simulink model "AcasXuClosedLoop_all_comp2017b"  (Simulink 9.0)
settings: Solver=VariableStepAuto  StartTime=0.0  StopTime=20  RelTol=1e-3
libraries: neural x14040, simulink x2
(part 26 of 534)

== system AcasXuClosedLoop_all_comp2017b/networks/Networks_m_6/AcasXu_2_6/Process Input 1 ==
  x : Inport  PortDimensions=5
      mask Display: e = 0.06;c1 = [120 230 180]/255;c2 = ([120 230 180]+0)/(256+128);c3 = ([120 230 180]+128)/(256+128);patch([0 1 1 0],[0 0 1 1],c1);patch([0 1 1 1-e 1-e 0+e],[0 0 1 1-e 0+e 0+e],c2);patch([0 0 1 1-e 0+e 0+e],[0 1 1 1-e 1-e 0+e],c3);plot([0 1 1 0 0],[0 0 1 1 0]);
  p : Outport  PortDimensions=5
      mask Display: e = 0.06;c1 = [120 230 180]/255;c2 = ([120 230 180]+0)/(256+128);c3 = ([120 230 180]+128)/(256+128);patch([0 1 1 0],[0 0 1 1],c1);patch([0 1 1 1-e 1-e 0+e],[0 0 1 1-e 0+e 0+e],c2);patch([0 0 1 1-e 0+e 0+e],[0 1 1 1-e 1-e 0+e],c3);plot([0 1 1 0 0],[0 0 1 1 0]);
  wires:
  x/1 -> p/1

== system AcasXuClosedLoop_all_comp2017b/networks/Networks_m_6/AcasXu_2_6/Process Output 1 ==
  a : Inport  PortDimensions=5
      mask Display: e = 0.06;c1 = [120 230 180]/255;c2 = ([120 230 180]+0)/(256+128);c3 = ([120 230 180]+128)/(256+128);patch([0 1 1 0],[0 0 1 1],c1);patch([0 1 1 1-e 1-e 0+e],[0 0 1 1-e 0+e 0+e],c2);patch([0 0 1 1-e 0+e 0+e],[0 1 1 1-e 1-e 0+e],c3);plot([0 1 1 0 0],[0 0 1 1 0]);
  y : Outport  PortDimensions=5
      mask Display: e = 0.06;c1 = [120 230 180]/255;c2 = ([120 230 180]+0)/(256+128);c3 = ([120 230 180]+128)/(256+128);patch([0 1 1 0],[0 0 1 1],c1);patch([0 1 1 1-e 1-e 0+e],[0 0 1 1-e 0+e 0+e],c2);patch([0 0 1 1-e 0+e 0+e],[0 1 1 1-e 1-e 0+e],c3);plot([0 1 1 0 0],[0 0 1 1 0]);
  wires:
  a/1 -> y/1

== system AcasXuClosedLoop_all_comp2017b/networks/Networks_m_6/AcasXu_3_6/Layer 1 ==
  "p{1}" : Inport  PortDimensions=5
      mask Display: e = 0.06;c1 = [120 230 180]/255;c2 = ([120 230 180]+0)/(256+128);c3 = ([120 230 180]+128)/(256+128);patch([0 1 1 0],[0 0 1 1],c1);patch([0 1 1 1-e 1-e 0+e],[0 0 1 1-e 0+e 0+e],c2);patch([0 0 1 1-e 0+e 0+e],[0 1 1 1-e 1-e 0+e],c3);plot([0 1 1 0 0],[0 0 1 1 0]);
  Delays 1 : Subsystem
      mask Display: e = 0.06;c1 = [120 150 230]/255;c2 = ([120 150 230]+0)/383;c3 = ([120 150 230]+128)/383;patch([0 1 1 0],[0 0 1 1],c1);patch([0 1 1 1-e 1-e 0+e],[0 0 1 1-e 0+e 0+e],c2);patch([0 0 1 1-e 0+e 0+e],[0 1 1 1-e 1-e 0+e],c3);plot([0 1 1 0 0],[0 0 1 1 0]);disp('0');
  "IW{1,1}" : Subsystem
      mask Display: e = 0.06;c1 = [120 150 230]/255;c2 = ([120 150 230]+0)/383;c3 = ([120 150 230]+128)/383;patch([0 1 1 0],[0 0 1 1],c1);patch([0 1 1 1-e 1-e 0+e],[0 0 1 1-e 0+e 0+e],c2);patch([0 0 1 1-e 0+e 0+e],[0 1 1 1-e 1-e 0+e],c3);plot([0 1 1 0 0],[0 0 1 1 0]);disp('W');
  "b{1}" : Constant  Value=[-0.04478279999999999760884605848332284949719905853271484375;-0.1006650000000000044764192352886311709880828857421875;-0.1662289999999999878799172847720910795032978057861328125;-0.114005999999999996230570786792668513953685760498046875;0.2104149999999999909316983348617213778197765350341796875;-0.1818849999999999911270975871957489289343357086181640625;-0.5026509999999999589448407277814112603664398193359375;0.7021830000000000016058265828178264200687408447265625;-0.270768999999999981920240088584250770509243011474609375;-0.09438190000000000468016736476783989928662776947021484375;0.0603599999999999969890751572165754623711109161376953125;-0.3025619999999999976125764078460633754730224609375;-0.05036960000000000048370196736868820153176784515380859375;0.01067079999999999938287142953186048544012010097503662109375;0.1083550000000000068656191842819680459797382354736328125;0.037607200000000000350564022255639429204165935516357421875;0.191655999999999993033128475872217677533626556396484375;-0.0214969999999999987483345620375985163263976573944091796875;0.08847919999999999396411709540188894607126712799072265625;-0.021716800000000001380495717739904648624360561370849609375;-0.4216670000000000140261136039043776690959930419921875;-0.300678000000000000824229573481716215610504150390625;-0.028699200000000001098410251643144874833524227142333984375;0.216254000000000001779909553079050965607166290283203125;-0.2151510000000000089048768359134555794298648834228515625;0.0309653000000000011571188451853231526911258697509765625;0.1150130000000000041193715105691808275878429412841796875;-0.2091349999999999875743839083952479995787143707275390625;0.0096367299999999996018562598010248620994389057159423828125;-0.67237700000000000244426701101474463939666748046875;-0.0248868999999999999939159778250541421584784984588623046875;0.135927999999999993274713006030651740729808807373046875;-0.380010000000000014441781104324036277830600738525390625;-0.0255956999999999990080823408789001405239105224609375;0.007737909999999999753617085929136010236106812953948974609375;-0.265739000000000002987832203871221281588077545166015625;0.1178990000000000037960745657983352430164813995361328125;-0.2221829999999999916138193611914175562560558319091796875;0.1361300000000000010036416142611415125429630279541015625;-0.0134143000000000005289546578524095821194350719451904296875;-0.1725250000000000116795462190566468052566051483154296875;0.05111580000000000290061308305666898377239704132080078125;-0.0088401000000000000411670697531008045189082622528076171875;-0.569296000000000024243718144134618341922760009765625;-0.227851999999999998980371174184256233274936676025390625;-0.407177999999999984392928809029399417340755462646484375;0.1075730000000000019522161665008752606809139251708984375;-0.28299099999999999255351212923415005207061767578125;-0.3689970000000000194262383956811390817165374755859375;0.038547199999999996744559638273130985908210277557373046875]
      mask Display: e = 0.06;c1 = [120 150 230]/255;c2 = ([120 150 230]+0)/383;c3 = ([120 150 230]+128)/383;patch([0 1 1 0],[0 0 1 1],c1);patch([0 1 1 1-e 1-e 0+e],[0 0 1 1-e 0+e 0+e],c2);patch([0 0 1 1-e 0+e 0+e],[0 1 1 1-e 1-e 0+e],c3);plot([0 1 1 0 0],[0 0 1 1 0]);disp('b');
  netsum : Sum  Inputs=2  SaturateOnIntegerOverflow=on  InputSameDT=on  OutDataTypeStr=Inherit: Same as first input
      mask Display: e = 0.06;c1 = [120 150 230]/255;c2 = ([120 150 230]+0)/383;c3 = ([120 150 230]+128)/383;patch([0 1 1 0],[0 0 1 1],c1);patch([0 1 1 1-e 1-e 0+e],[0 0 1 1-e 0+e 0+e],c2);patch([0 0 1 1-e 0+e 0+e],[0 1 1 1-e 1-e 0+e],c3);plot([0 1 1 0 0],[0 0 1 1 0]);disp('+');
  poslin : neural/Transfer Functions/poslin
      settings: SourceProductName=Deep Learning Toolbox  SourceProductBaseCode=NN
  "a{1}" : Outport  InitialOutput=[0;0;0;0;0;0;0;0;0;0;0;0;0;0;0;0;0;0;0;0;0;0;0;0;0;0;0;0;0;0;0;0;0;0;0;0;0;0;0;0;0;0;0;0;0;0;0;0;0;0]
      mask Display: e = 0.06;c1 = [120 230 180]/255;c2 = ([120 230 180]+0)/(256+128);c3 = ([120 230 180]+128)/(256+128);patch([0 1 1 0],[0 0 1 1],c1);patch([0 1 1 1-e 1-e 0+e],[0 0 1 1-e 0+e 0+e],c2);patch([0 0 1 1-e 0+e 0+e],[0 1 1 1-e 1-e 0+e],c3);plot([0 1 1 0 0],[0 0 1 1 0]);
  wires:
  poslin/1 -> "a{1}"/1
  netsum/1 -> poslin/1
  "b{1}"/1 -> netsum/2
  "IW{1,1}"/1("iz{1,1}") -> netsum/1
  Delays 1/1("pd{1,1}") -> "IW{1,1}"/1("pd{1,1}")
  "p{1}"/1 -> Delays 1/1("p{1}")

== system AcasXuClosedLoop_all_comp2017b/networks/Networks_m_6/AcasXu_3_6/Layer 2 ==
  "a{1} " : Inport  PortDimensions=50
      mask Display: e = 0.06;c1 = [120 230 180]/255;c2 = ([120 230 180]+0)/(256+128);c3 = ([120 230 180]+128)/(256+128);patch([0 1 1 0],[0 0 1 1],c1);patch([0 1 1 1-e 1-e 0+e],[0 0 1 1-e 0+e 0+e],c2);patch([0 0 1 1-e 0+e 0+e],[0 1 1 1-e 1-e 0+e],c3);plot([0 1 1 0 0],[0 0 1 1 0]);
  Delays 1 : Subsystem
      mask Display: e = 0.06;c1 = [120 150 230]/255;c2 = ([120 150 230]+0)/383;c3 = ([120 150 230]+128)/383;patch([0 1 1 0],[0 0 1 1],c1);patch([0 1 1 1-e 1-e 0+e],[0 0 1 1-e 0+e 0+e],c2);patch([0 0 1 1-e 0+e 0+e],[0 1 1 1-e 1-e 0+e],c3);plot([0 1 1 0 0],[0 0 1 1 0]);disp('0');
  "LW{2,1}" : Subsystem
      mask Display: e = 0.06;c1 = [120 150 230]/255;c2 = ([120 150 230]+0)/383;c3 = ([120 150 230]+128)/383;patch([0 1 1 0],[0 0 1 1],c1);patch([0 1 1 1-e 1-e 0+e],[0 0 1 1-e 0+e 0+e],c2);patch([0 0 1 1-e 0+e 0+e],[0 1 1 1-e 1-e 0+e],c3);plot([0 1 1 0 0],[0 0 1 1 0]);disp('W');
  "b{2}" : Constant  Value=[-0.715594999999999981099563228781335055828094482421875;0.22643299999999999538857764491694979369640350341796875;0.46773399999999998311039917098241858184337615966796875;-0.02307309999999999916564519253370235674083232879638671875;-0.99585800000000002096811613228055648505687713623046875;-0.532162000000000023902657630969770252704620361328125;0.6978239999999999998436805981327779591083526611328125;0.315493000000000023419488570652902126312255859375;-0.03022110000000000074038553066202439367771148681640625;-0.0299515999999999983638421241494143032468855381011962890625;-0.313103999999999993431032407897873781621456146240234375;-0.881032999999999955065277390531264245510101318359375;-0.187649000000000010235368108624243177473545074462890625;-2.010170000000000012363443602225743234157562255859375;-0.020385500000000000841993141875718720257282257080078125;-0.260166999999999981607601284849806688725948333740234375;0.3023759999999999781294945933041162788867950439453125;-0.0087849699999999995847499434376004501245915889739990234375;-0.01171920000000000079698470045741487410850822925567626953125;1.3038199999999999789679350215010344982147216796875;-0.10043699999999999850075482754618860781192779541015625;-0.486456999999999972761344224636559374630451202392578125;0.2458220000000000127204913269451935775578022003173828125;-0.24828200000000000269295696853077970445156097412109375;1.10846000000000000085265128291212022304534912109375;-0.11688300000000000078426154459521058015525341033935546875;-0.126939999999999997282174035717616789042949676513671875;-0.039563700000000000256417109767426154576241970062255859375;0.310024000000000021781687564725871197879314422607421875;-0.00080762900000000002916078489789697414380498230457305908203125;0.623121999999999953701035337871871888637542724609375;0.046389199999999998491606589823277317918837070465087890625;0.2120519999999999904982672660480602644383907318115234375;-0.0149935999999999992893240374769447953440248966217041015625;-0.09922240000000000226254570634409901686012744903564453125;0.0916484999999999938591344061933341436088085174560546875;0.373078999999999993963939459717948921024799346923828125;-0.038554600000000001369659941019563120789825916290283203125;0.1619210000000000093667296141575207002460956573486328125;-0.01232910000000000082243101218182346201501786708831787109375;-0.059849600000000002797406750687514431774616241455078125;-0.296686999999999978516740384293370880186557769775390625;-1.0887000000000000010658141036401502788066864013671875;-0.08855090000000000183266735120923840440809726715087890625;0.36397000000000001573852159708621911704540252685546875;0.235756999999999994344079823349602520465850830078125;-0.021244800000000001183320108566476847045123577117919921875;1.6311299999999999688071739001316018402576446533203125;-0.164617999999999986560084153097704984247684478759765625;0.0197745999999999998164579295689691207371652126312255859375]
      mask Display: e = 0.06;c1 = [120 150 230]/255;c2 = ([120 150 230]+0)/383;c3 = ([120 150 230]+128)/383;patch([0 1 1 0],[0 0 1 1],c1);patch([0 1 1 1-e 1-e 0+e],[0 0 1 1-e 0+e 0+e],c2);patch([0 0 1 1-e 0+e 0+e],[0 1 1 1-e 1-e 0+e],c3);plot([0 1 1 0 0],[0 0 1 1 0]);disp('b');
  netsum : Sum  Inputs=2  SaturateOnIntegerOverflow=on  InputSameDT=on  OutDataTypeStr=Inherit: Same as first input
      mask Display: e = 0.06;c1 = [120 150 230]/255;c2 = ([120 150 230]+0)/383;c3 = ([120 150 230]+128)/383;patch([0 1 1 0],[0 0 1 1],c1);patch([0 1 1 1-e 1-e 0+e],[0 0 1 1-e 0+e 0+e],c2);patch([0 0 1 1-e 0+e 0+e],[0 1 1 1-e 1-e 0+e],c3);plot([0 1 1 0 0],[0 0 1 1 0]);disp('+');
  poslin : neural/Transfer Functions/poslin
      settings: SourceProductName=Deep Learning Toolbox  SourceProductBaseCode=NN
  "a{2}" : Outport  InitialOutput=[0;0;0;0;0;0;0;0;0;0;0;0;0;0;0;0;0;0;0;0;0;0;0;0;0;0;0;0;0;0;0;0;0;0;0;0;0;0;0;0;0;0;0;0;0;0;0;0;0;0]
      mask Display: e = 0.06;c1 = [120 230 180]/255;c2 = ([120 230 180]+0)/(256+128);c3 = ([120 230 180]+128)/(256+128);patch([0 1 1 0],[0 0 1 1],c1);patch([0 1 1 1-e 1-e 0+e],[0 0 1 1-e 0+e 0+e],c2);patch([0 0 1 1-e 0+e 0+e],[0 1 1 1-e 1-e 0+e],c3);plot([0 1 1 0 0],[0 0 1 1 0]);
  wires:
  poslin/1 -> "a{2}"/1
  netsum/1 -> poslin/1
  "b{2}"/1 -> netsum/2
  "LW{2,1}"/1("lz{2,1}") -> netsum/1
  Delays 1/1("pd{2,1}") -> "LW{2,1}"/1("ad{2,1}")
  "a{1} "/1 -> Delays 1/1("p{2}")

== system AcasXuClosedLoop_all_comp2017b/networks/Networks_m_6/AcasXu_3_6/Layer 3 ==
  "a{2} " : Inport  PortDimensions=50
      mask Display: e = 0.06;c1 = [120 230 180]/255;c2 = ([120 230 180]+0)/(256+128);c3 = ([120 230 180]+128)/(256+128);patch([0 1 1 0],[0 0 1 1],c1);patch([0 1 1 1-e 1-e 0+e],[0 0 1 1-e 0+e 0+e],c2);patch([0 0 1 1-e 0+e 0+e],[0 1 1 1-e 1-e 0+e],c3);plot([0 1 1 0 0],[0 0 1 1 0]);
  Delays 1 : Subsystem
      mask Display: e = 0.06;c1 = [120 150 230]/255;c2 = ([120 150 230]+0)/383;c3 = ([120 150 230]+128)/383;patch([0 1 1 0],[0 0 1 1],c1);patch([0 1 1 1-e 1-e 0+e],[0 0 1 1-e 0+e 0+e],c2);patch([0 0 1 1-e 0+e 0+e],[0 1 1 1-e 1-e 0+e],c3);plot([0 1 1 0 0],[0 0 1 1 0]);disp('0');
  "LW{3,2}" : Subsystem
      mask Display: e = 0.06;c1 = [120 150 230]/255;c2 = ([120 150 230]+0)/383;c3 = ([120 150 230]+128)/383;patch([0 1 1 0],[0 0 1 1],c1);patch([0 1 1 1-e 1-e 0+e],[0 0 1 1-e 0+e 0+e],c2);patch([0 0 1 1-e 0+e 0+e],[0 1 1 1-e 1-e 0+e],c3);plot([0 1 1 0 0],[0 0 1 1 0]);disp('W');
  "b{3}" : Constant  Value=[0.6864200000000000301270119962282478809356689453125;-1.2543599999999999194955080383806489408016204833984375;0.8977079999999999504467496080906130373477935791015625;0.0270840000000000004354294702579863951541483402252197265625;-0.5959170000000000300133251585066318511962890625;0.88390899999999994474109143993700854480266571044921875;-0.72104500000000004700240197053062729537487030029296875;-0.5280860000000000553654899704270064830780029296875;-0.65206299999999994820853999044629745185375213623046875;-2.50091999999999980985876391059719026088714599609375;-0.96922900000000000719779791324981488287448883056640625;0.213511000000000006338041202980093657970428466796875;-0.035338899999999999479438628213756601326167583465576171875;1.501279999999999947846163195208646357059478759765625;-1.445629999999999970583530739531852304935455322265625;-0.77240900000000001224265133714652620255947113037109375;1.2072499999999999342747969421907328069210052490234375;-1.0386599999999999166533370953402481973171234130859375;1.277179999999999981952214511693455278873443603515625;-1.5873399999999999732125388618442229926586151123046875;-0.62160899999999996712318761638016439974308013916015625;-0.5893770000000000397477606384200043976306915283203125;-0.1674980000000000079918294204617268405854701995849609375;0.1195819999999999938555816925145336426794528961181640625;-0.0120474999999999991817656308512596297077834606170654296875;-1.0430900000000000726885218682582490146160125732421875;0.342283999999999977159603758991579525172710418701171875;-0.191597999999999990539123473354266025125980377197265625;-1.8245999999999999996447286321199499070644378662109375;0.512985000000000024300561562995426356792449951171875;-0.4961349999999999926814098216709680855274200439453125;-1.4075200000000001043787278831587173044681549072265625;0.1018840000000000023394619574901298619806766510009765625;0.85067499999999995896615700985421426594257354736328125;0.43413299999999999112532123035634867846965789794921875;-0.12126399999999999679278772646284778602421283721923828125;-0.50857699999999994577848383414675481617450714111328125;0.012540900000000000658051391155822784639894962310791015625;0.1964430000000000064996896753655164502561092376708984375;-0.2051510000000000000230926389122032560408115386962890625;-0.058999799999999998079136531714539160020649433135986328125;-2.221470000000000055706550483591854572296142578125;-0.53654400000000002091127271341974847018718719482421875;-0.60596300000000002938804755103774368762969970703125;-1.097469999999999945572426440776325762271881103515625;-0.84963800000000000434141611549421213567256927490234375;-0.1586490000000000122337695529495249502360820770263671875;-0.2483559999999999934328087647372740320861339569091796875;-0.087763599999999997169908283467520959675312042236328125;0.2608289999999999775326386952656321227550506591796875]
      mask Display: e = 0.06;c1 = [120 150 230]/255;c2 = ([120 150 230]+0)/383;c3 = ([120 150 230]+128)/383;patch([0 1 1 0],[0 0 1 1],c1);patch([0 1 1 1-e 1-e 0+e],[0 0 1 1-e 0+e 0+e],c2);patch([0 0 1 1-e 0+e 0+e],[0 1 1 1-e 1-e 0+e],c3);plot([0 1 1 0 0],[0 0 1 1 0]);disp('b');
  netsum : Sum  Inputs=2  SaturateOnIntegerOverflow=on  InputSameDT=on  OutDataTypeStr=Inherit: Same as first input
      mask Display: e = 0.06;c1 = [120 150 230]/255;c2 = ([120 150 230]+0)/383;c3 = ([120 150 230]+128)/383;patch([0 1 1 0],[0 0 1 1],c1);patch([0 1 1 1-e 1-e 0+e],[0 0 1 1-e 0+e 0+e],c2);patch([0 0 1 1-e 0+e 0+e],[0 1 1 1-e 1-e 0+e],c3);plot([0 1 1 0 0],[0 0 1 1 0]);disp('+');
  poslin : neural/Transfer Functions/poslin
      settings: SourceProductName=Deep Learning Toolbox  SourceProductBaseCode=NN
  "a{3}" : Outport  InitialOutput=[0;0;0;0;0;0;0;0;0;0;0;0;0;0;0;0;0;0;0;0;0;0;0;0;0;0;0;0;0;0;0;0;0;0;0;0;0;0;0;0;0;0;0;0;0;0;0;0;0;0]
      mask Display: e = 0.06;c1 = [120 230 180]/255;c2 = ([120 230 180]+0)/(256+128);c3 = ([120 230 180]+128)/(256+128);patch([0 1 1 0],[0 0 1 1],c1);patch([0 1 1 1-e 1-e 0+e],[0 0 1 1-e 0+e 0+e],c2);patch([0 0 1 1-e 0+e 0+e],[0 1 1 1-e 1-e 0+e],c3);plot([0 1 1 0 0],[0 0 1 1 0]);
  wires:
  poslin/1 -> "a{3}"/1
  netsum/1 -> poslin/1
  "b{3}"/1 -> netsum/2
  "LW{3,2}"/1("lz{3,2}") -> netsum/1
  Delays 1/1("pd{3,2}") -> "LW{3,2}"/1("ad{3,2}")
  "a{2} "/1 -> Delays 1/1("p{3}")

== system AcasXuClosedLoop_all_comp2017b/networks/Networks_m_6/AcasXu_3_6/Layer 4 ==
  "a{3} " : Inport  PortDimensions=50
      mask Display: e = 0.06;c1 = [120 230 180]/255;c2 = ([120 230 180]+0)/(256+128);c3 = ([120 230 180]+128)/(256+128);patch([0 1 1 0],[0 0 1 1],c1);patch([0 1 1 1-e 1-e 0+e],[0 0 1 1-e 0+e 0+e],c2);patch([0 0 1 1-e 0+e 0+e],[0 1 1 1-e 1-e 0+e],c3);plot([0 1 1 0 0],[0 0 1 1 0]);
  Delays 1 : Subsystem
      mask Display: e = 0.06;c1 = [120 150 230]/255;c2 = ([120 150 230]+0)/383;c3 = ([120 150 230]+128)/383;patch([0 1 1 0],[0 0 1 1],c1);patch([0 1 1 1-e 1-e 0+e],[0 0 1 1-e 0+e 0+e],c2);patch([0 0 1 1-e 0+e 0+e],[0 1 1 1-e 1-e 0+e],c3);plot([0 1 1 0 0],[0 0 1 1 0]);disp('0');
  "LW{4,3}" : Subsystem
      mask Display: e = 0.06;c1 = [120 150 230]/255;c2 = ([120 150 230]+0)/383;c3 = ([120 150 230]+128)/383;patch([0 1 1 0],[0 0 1 1],c1);patch([0 1 1 1-e 1-e 0+e],[0 0 1 1-e 0+e 0+e],c2);patch([0 0 1 1-e 0+e 0+e],[0 1 1 1-e 1-e 0+e],c3);plot([0 1 1 0 0],[0 0 1 1 0]);disp('W');
  "b{4}" : Constant  Value=[1.5520300000000000206767936106189154088497161865234375;-0.70960199999999995501553939902805723249912261962890625;2.935770000000000212736495086573995649814605712890625;-3.54572000000000020492052499321289360523223876953125;0.2113420000000000020801138589376932941377162933349609375;1.093949999999999977973175191436894237995147705078125;-0.08387930000000000385629306265400373376905918121337890625;3.480379999999999807158701514708809554576873779296875;0.72688099999999999933919525574310682713985443115234375;0.97945099999999996054356188324163667857646942138671875;-1.1935100000000000708411107552819885313510894775390625;0.87588999999999994638955058690044097602367401123046875;-2.689150000000000151345602716901339590549468994140625;1.212450000000000027711166694643907248973846435546875;-1.9826799999999999979394260662957094609737396240234375;2.1572800000000000864019966684281826019287109375;-0.67117400000000004833822231375961564481258392333984375;0.368535000000000001474376176702207885682582855224609375;2.669189999999999951540985421161167323589324951171875;-0.2201700000000000045918824298496474511921405792236328125;-4.827890000000000014779288903810083866119384765625;1.2511799999999999588595755994901992380619049072265625;0.40600300000000000277822209682199172675609588623046875;-1.4874199999999999644018089384189806878566741943359375;-0.0244275000000000012956302697375576826743781566619873046875;3.127569999999999961204366627498529851436614990234375;-1.2717199999999999615596379953785799443721771240234375;0.584025000000000016342482922482304275035858154296875;4.71075999999999961431740302941761910915374755859375;1.5830200000000000937205868467572145164012908935546875;-0.78743700000000005356781684895395301282405853271484375;-0.0159807999999999998663735567561161587946116924285888671875;2.29061000000000003495870259939692914485931396484375;-0.88526899999999997259436668173293583095073699951171875;-0.6403149999999999675281969757634215056896209716796875;0.36229400000000000492406115881749428808689117431640625;0.232202999999999992741805954210576601326465606689453125;-3.775650000000000172661884789704345166683197021484375;-0.7711770000000000013784529073745943605899810791015625;-1.866800000000000014921397450962103903293609619140625;0.482495999999999980456522052918444387614727020263671875;-0.97538999999999997925215211580507457256317138671875;0.82735199999999997633182147183106280863285064697265625;-1.5931100000000000260769184023956768214702606201171875;-0.08347590000000000565183455591977690346539020538330078125;-0.1837249999999999994226840271949185989797115325927734375;0.66559500000000004771294470629072748124599456787109375;1.4274299999999999766231439934927038848400115966796875;2.28727999999999997982058630441315472126007080078125;-1.3732699999999999906918901615426875650882720947265625]
      mask Display: e = 0.06;c1 = [120 150 230]/255;c2 = ([120 150 230]+0)/383;c3 = ([120 150 230]+128)/383;patch([0 1 1 0],[0 0 1 1],c1);patch([0 1 1 1-e 1-e 0+e],[0 0 1 1-e 0+e 0+e],c2);patch([0 0 1 1-e 0+e 0+e],[0 1 1 1-e 1-e 0+e],c3);plot([0 1 1 0 0],[0 0 1 1 0]);disp('b');
  netsum : Sum  Inputs=2  SaturateOnIntegerOverflow=on  InputSameDT=on  OutDataTypeStr=Inherit: Same as first input
      mask Display: e = 0.06;c1 = [120 150 230]/255;c2 = ([120 150 230]+0)/383;c3 = ([120 150 230]+128)/383;patch([0 1 1 0],[0 0 1 1],c1);patch([0 1 1 1-e 1-e 0+e],[0 0 1 1-e 0+e 0+e],c2);patch([0 0 1 1-e 0+e 0+e],[0 1 1 1-e 1-e 0+e],c3);plot([0 1 1 0 0],[0 0 1 1 0]);disp('+');
  poslin : neural/Transfer Functions/poslin
      settings: SourceProductName=Deep Learning Toolbox  SourceProductBaseCode=NN
  "a{4}" : Outport  InitialOutput=[0;0;0;0;0;0;0;0;0;0;0;0;0;0;0;0;0;0;0;0;0;0;0;0;0;0;0;0;0;0;0;0;0;0;0;0;0;0;0;0;0;0;0;0;0;0;0;0;0;0]
      mask Display: e = 0.06;c1 = [120 230 180]/255;c2 = ([120 230 180]+0)/(256+128);c3 = ([120 230 180]+128)/(256+128);patch([0 1 1 0],[0 0 1 1],c1);patch([0 1 1 1-e 1-e 0+e],[0 0 1 1-e 0+e 0+e],c2);patch([0 0 1 1-e 0+e 0+e],[0 1 1 1-e 1-e 0+e],c3);plot([0 1 1 0 0],[0 0 1 1 0]);
  wires:
  poslin/1 -> "a{4}"/1
  netsum/1 -> poslin/1
  "b{4}"/1 -> netsum/2
  "LW{4,3}"/1("lz{4,3}") -> netsum/1
  Delays 1/1("pd{4,3}") -> "LW{4,3}"/1("ad{4,3}")
  "a{3} "/1 -> Delays 1/1("p{4}")

== system AcasXuClosedLoop_all_comp2017b/networks/Networks_m_6/AcasXu_3_6/Layer 5 ==
  "a{4} " : Inport  PortDimensions=50
      mask Display: e = 0.06;c1 = [120 230 180]/255;c2 = ([120 230 180]+0)/(256+128);c3 = ([120 230 180]+128)/(256+128);patch([0 1 1 0],[0 0 1 1],c1);patch([0 1 1 1-e 1-e 0+e],[0 0 1 1-e 0+e 0+e],c2);patch([0 0 1 1-e 0+e 0+e],[0 1 1 1-e 1-e 0+e],c3);plot([0 1 1 0 0],[0 0 1 1 0]);
  Delays 1 : Subsystem
      mask Display: e = 0.06;c1 = [120 150 230]/255;c2 = ([120 150 230]+0)/383;c3 = ([120 150 230]+128)/383;patch([0 1 1 0],[0 0 1 1],c1);patch([0 1 1 1-e 1-e 0+e],[0 0 1 1-e 0+e 0+e],c2);patch([0 0 1 1-e 0+e 0+e],[0 1 1 1-e 1-e 0+e],c3);plot([0 1 1 0 0],[0 0 1 1 0]);disp('0');
  "LW{5,4}" : Subsystem
      mask Display: e = 0.06;c1 = [120 150 230]/255;c2 = ([120 150 230]+0)/383;c3 = ([120 150 230]+128)/383;patch([0 1 1 0],[0 0 1 1],c1);patch([0 1 1 1-e 1-e 0+e],[0 0 1 1-e 0+e 0+e],c2);patch([0 0 1 1-e 0+e 0+e],[0 1 1 1-e 1-e 0+e],c3);plot([0 1 1 0 0],[0 0 1 1 0]);disp('W');
  "b{5}" : Constant  Value=[1.5628599999999999159427943595801480114459991455078125;0.453656000000000003691269512273720465600490570068359375;-1.8718999999999998973265746826655231416225433349609375;-0.65323600000000003884537136400467716157436370849609375;2.4535999999999997811528373858891427516937255859375;-1.114640000000000075175421443418599665164947509765625;1.89552999999999993718802215880714356899261474609375;-0.3666519999999999779305426272912882268428802490234375;-0.07712479999999999336068867705762386322021484375;1.6370400000000000506616970596951432526111602783203125;-2.1789100000000001244870873051695525646209716796875;0.3260009999999999852349219509051181375980377197265625;0.6327540000000000386393139706342481076717376708984375;0.2375279999999999891446833544250694103538990020751953125;3.057939999999999880486711845151148736476898193359375;3.81092999999999992866150932968594133853912353515625;-0.22313199999999999700861508244997821748256683349609375;-0.453898999999999996912691813122364692389965057373046875;0.73527500000000001190159082398167811334133148193359375;4.12155999999999966831865094718523323535919189453125;0.87841199999999997061195244896225631237030029296875;-0.2744750000000000245137243837234564125537872314453125;3.16481000000000012306600183364935219287872314453125;-1.422940000000000093649532573181204497814178466796875;0.27830100000000002058442305497010238468647003173828125;0.11552400000000000168665081901053781621158123016357421875;1.4645200000000000439825953435502015054225921630859375;0.24853300000000000391509047403815202414989471435546875;2.818449999999999899813474257825873792171478271484375;-0.0235788000000000004530154029680488747544586658477783203125;-0.945319000000000020378365661599673330783843994140625;-0.881345000000000045048409447190351784229278564453125;3.185379999999999878212975090718828141689300537109375;1.6038300000000000888888962435885332524776458740234375;-0.04964369999999999893969260256199049763381481170654296875;1.3275600000000000733280103304423391819000244140625;0.736423999999999967513986121048219501972198486328125;0.1944559999999999899689129279067856259644031524658203125;-2.629449999999999842970055397017858922481536865234375;-2.539429999999999854054522074875421822071075439453125;1.3961300000000000931521526581491343677043914794921875;0.0671940000000000037250202922223252244293689727783203125;-0.046682200000000000084110496345601859502494335174560546875;6.34015000000000039648284655413590371608734130859375;1.753390000000000004121147867408581078052520751953125;0.670112000000000040955683289212174713611602783203125;3.29307000000000016370904631912708282470703125;-0.53057600000000004758504701385390944778919219970703125;-0.3941069999999999851070242584683001041412353515625;0.052505299999999997806465756866600713692605495452880859375]
      mask Display: e = 0.06;c1 = [120 150 230]/255;c2 = ([120 150 230]+0)/383;c3 = ([120 150 230]+128)/383;patch([0 1 1 0],[0 0 1 1],c1);patch([0 1 1 1-e 1-e 0+e],[0 0 1 1-e 0+e 0+e],c2);patch([0 0 1 1-e 0+e 0+e],[0 1 1 1-e 1-e 0+e],c3);plot([0 1 1 0 0],[0 0 1 1 0]);disp('b');
  netsum : Sum  Inputs=2  SaturateOnIntegerOverflow=on  InputSameDT=on  OutDataTypeStr=Inherit: Same as first input
      mask Display: e = 0.06;c1 = [120 150 230]/255;c2 = ([120 150 230]+0)/383;c3 = ([120 150 230]+128)/383;patch([0 1 1 0],[0 0 1 1],c1);patch([0 1 1 1-e 1-e 0+e],[0 0 1 1-e 0+e 0+e],c2);patch([0 0 1 1-e 0+e 0+e],[0 1 1 1-e 1-e 0+e],c3);plot([0 1 1 0 0],[0 0 1 1 0]);disp('+');
  poslin : neural/Transfer Functions/poslin
      settings: SourceProductName=Deep Learning Toolbox  SourceProductBaseCode=NN
  "a{5}" : Outport  InitialOutput=[0;0;0;0;0;0;0;0;0;0;0;0;0;0;0;0;0;0;0;0;0;0;0;0;0;0;0;0;0;0;0;0;0;0;0;0;0;0;0;0;0;0;0;0;0;0;0;0;0;0]
      mask Display: e = 0.06;c1 = [120 230 180]/255;c2 = ([120 230 180]+0)/(256+128);c3 = ([120 230 180]+128)/(256+128);patch([0 1 1 0],[0 0 1 1],c1);patch([0 1 1 1-e 1-e 0+e],[0 0 1 1-e 0+e 0+e],c2);patch([0 0 1 1-e 0+e 0+e],[0 1 1 1-e 1-e 0+e],c3);plot([0 1 1 0 0],[0 0 1 1 0]);
  wires:
  poslin/1 -> "a{5}"/1
  netsum/1 -> poslin/1
  "b{5}"/1 -> netsum/2
  "LW{5,4}"/1("lz{5,4}") -> netsum/1
  Delays 1/1("pd{5,4}") -> "LW{5,4}"/1("ad{5,4}")
  "a{4} "/1 -> Delays 1/1("p{5}")

== system AcasXuClosedLoop_all_comp2017b/networks/Networks_m_6/AcasXu_3_6/Layer 6 ==
  "a{5} " : Inport  PortDimensions=50
      mask Display: e = 0.06;c1 = [120 230 180]/255;c2 = ([120 230 180]+0)/(256+128);c3 = ([120 230 180]+128)/(256+128);patch([0 1 1 0],[0 0 1 1],c1);patch([0 1 1 1-e 1-e 0+e],[0 0 1 1-e 0+e 0+e],c2);patch([0 0 1 1-e 0+e 0+e],[0 1 1 1-e 1-e 0+e],c3);plot([0 1 1 0 0],[0 0 1 1 0]);
  Delays 1 : Subsystem
      mask Display: e = 0.06;c1 = [120 150 230]/255;c2 = ([120 150 230]+0)/383;c3 = ([120 150 230]+128)/383;patch([0 1 1 0],[0 0 1 1],c1);patch([0 1 1 1-e 1-e 0+e],[0 0 1 1-e 0+e 0+e],c2);patch([0 0 1 1-e 0+e 0+e],[0 1 1 1-e 1-e 0+e],c3);plot([0 1 1 0 0],[0 0 1 1 0]);disp('0');
  "LW{6,5}" : Subsystem
      mask Display: e = 0.06;c1 = [120 150 230]/255;c2 = ([120 150 230]+0)/383;c3 = ([120 150 230]+128)/383;patch([0 1 1 0],[0 0 1 1],c1);patch([0 1 1 1-e 1-e 0+e],[0 0 1 1-e 0+e 0+e],c2);patch([0 0 1 1-e 0+e 0+e],[0 1 1 1-e 1-e 0+e],c3);plot([0 1 1 0 0],[0 0 1 1 0]);disp('W');
  "b{6}" : Constant  Value=[0.39295000000000002149391775674303062260150909423828125;0.9977380000000000137561073643155395984649658203125;0.7563940000000000107860387288383208215236663818359375;0.2563750000000000195399252334027551114559173583984375;-1.3063700000000000311928260998683981597423553466796875;-0.53875399999999995515054251882247626781463623046875;1.3839900000000000535038680027355439960956573486328125;1.4431599999999999983657517077517695724964141845703125;-2.615159999999999929087834971142001450061798095703125;0.65935299999999996689581394093693234026432037353515625;0.5012760000000000548681100553949363529682159423828125;3.209630000000000205062633540364913642406463623046875;-3.070769999999999999573674358543939888477325439453125;-0.1127380000000000048743231673142872750759124755859375;-0.71589000000000002632560835991171188652515411376953125;-2.716769999999999907203118709730915725231170654296875;1.942509999999999958930629873066209256649017333984375;-1.08051999999999992496668710373342037200927734375;-1.48388000000000008782308213994838297367095947265625;0.450102999999999975333508928088122047483921051025390625;1.523779999999999912319026407203637063503265380859375;1.7611200000000000187583282240666449069976806640625;3.966250000000000053290705182007513940334320068359375;0.505117999999999955917928673443384468555450439453125;1.7869900000000000783728637543390505015850067138671875;-4.10649999999999959499064061674289405345916748046875;2.338010000000000143671741170692257583141326904296875;0.92881599999999997496757941917167045176029205322265625;1.3119799999999999240429815472452901303768157958984375;0.6370489999999999763957703180494718253612518310546875;-0.1291569999999999940332173764545586891472339630126953125;1.22951999999999994628296917653642594814300537109375;0.035326799999999998480593177418995765037834644317626953125;1.0600199999999999622701807311386801302433013916015625;0.30689800000000000412825329476618207991123199462890625;2.6040299999999998448174665099941194057464599609375;1.0950699999999999878497192185022868216037750244140625;0.3489240000000000119229071060544811189174652099609375;0.658139999999999947277729006600566208362579345703125;1.7974099999999999521804738833452574908733367919921875;1.2925599999999999312194631784223020076751708984375;-0.90671400000000001995204002014361321926116943359375;-0.479970999999999980989429104738519527018070220947265625;2.797239999999999948698814478120766580104827880859375;-0.64321499999999998120614463914535008370876312255859375;0.296623999999999998777866494492627680301666259765625;1.091159999999999907771552898338995873928070068359375;-2.925660000000000149356083056773059070110321044921875;-0.94354899999999997106669979984872043132781982421875;0.050657399999999998263522371644285158254206180572509765625]
      mask Display: e = 0.06;c1 = [120 150 230]/255;c2 = ([120 150 230]+0)/383;c3 = ([120 150 230]+128)/383;patch([0 1 1 0],[0 0 1 1],c1);patch([0 1 1 1-e 1-e 0+e],[0 0 1 1-e 0+e 0+e],c2);patch([0 0 1 1-e 0+e 0+e],[0 1 1 1-e 1-e 0+e],c3);plot([0 1 1 0 0],[0 0 1 1 0]);disp('b');
  netsum : Sum  Inputs=2  SaturateOnIntegerOverflow=on  InputSameDT=on  OutDataTypeStr=Inherit: Same as first input
      mask Display: e = 0.06;c1 = [120 150 230]/255;c2 = ([120 150 230]+0)/383;c3 = ([120 150 230]+128)/383;patch([0 1 1 0],[0 0 1 1],c1);patch([0 1 1 1-e 1-e 0+e],[0 0 1 1-e 0+e 0+e],c2);patch([0 0 1 1-e 0+e 0+e],[0 1 1 1-e 1-e 0+e],c3);plot([0 1 1 0 0],[0 0 1 1 0]);disp('+');
  poslin : neural/Transfer Functions/poslin
      settings: SourceProductName=Deep Learning Toolbox  SourceProductBaseCode=NN
  "a{6}" : Outport  InitialOutput=[0;0;0;0;0;0;0;0;0;0;0;0;0;0;0;0;0;0;0;0;0;0;0;0;0;0;0;0;0;0;0;0;0;0;0;0;0;0;0;0;0;0;0;0;0;0;0;0;0;0]
      mask Display: e = 0.06;c1 = [120 230 180]/255;c2 = ([120 230 180]+0)/(256+128);c3 = ([120 230 180]+128)/(256+128);patch([0 1 1 0],[0 0 1 1],c1);patch([0 1 1 1-e 1-e 0+e],[0 0 1 1-e 0+e 0+e],c2);patch([0 0 1 1-e 0+e 0+e],[0 1 1 1-e 1-e 0+e],c3);plot([0 1 1 0 0],[0 0 1 1 0]);
  wires:
  poslin/1 -> "a{6}"/1
  netsum/1 -> poslin/1
  "b{6}"/1 -> netsum/2
  "LW{6,5}"/1("lz{6,5}") -> netsum/1
  Delays 1/1("pd{6,5}") -> "LW{6,5}"/1("ad{6,5}")
  "a{5} "/1 -> Delays 1/1("p{6}")

== system AcasXuClosedLoop_all_comp2017b/networks/Networks_m_6/AcasXu_3_6/Layer 7 ==
  "a{6} " : Inport  PortDimensions=50
      mask Display: e = 0.06;c1 = [120 230 180]/255;c2 = ([120 230 180]+0)/(256+128);c3 = ([120 230 180]+128)/(256+128);patch([0 1 1 0],[0 0 1 1],c1);patch([0 1 1 1-e 1-e 0+e],[0 0 1 1-e 0+e 0+e],c2);patch([0 0 1 1-e 0+e 0+e],[0 1 1 1-e 1-e 0+e],c3);plot([0 1 1 0 0],[0 0 1 1 0]);
  Delays 1 : Subsystem
      mask Display: e = 0.06;c1 = [120 150 230]/255;c2 = ([120 150 230]+0)/383;c3 = ([120 150 230]+128)/383;patch([0 1 1 0],[0 0 1 1],c1);patch([0 1 1 1-e 1-e 0+e],[0 0 1 1-e 0+e 0+e],c2);patch([0 0 1 1-e 0+e 0+e],[0 1 1 1-e 1-e 0+e],c3);plot([0 1 1 0 0],[0 0 1 1 0]);disp('0');
  "LW{7,6}" : Subsystem
      mask Display: e = 0.06;c1 = [120 150 230]/255;c2 = ([120 150 230]+0)/383;c3 = ([120 150 230]+128)/383;patch([0 1 1 0],[0 0 1 1],c1);patch([0 1 1 1-e 1-e 0+e],[0 0 1 1-e 0+e 0+e],c2);patch([0 0 1 1-e 0+e 0+e],[0 1 1 1-e 1-e 0+e],c3);plot([0 1 1 0 0],[0 0 1 1 0]);disp('W');
  "b{7}" : Constant  Value=[-0.0195876000000000001499689261663661454804241657257080078125;0.0187767999999999997406963103685484384186565876007080078125;-0.02017170000000000074091843771384446881711483001708984375;0.019597599999999999742517076128933695144951343536376953125;-0.01782419999999999837836384131151135079562664031982421875]
      mask Display: e = 0.06;c1 = [120 150 230]/255;c2 = ([120 150 230]+0)/383;c3 = ([120 150 230]+128)/383;patch([0 1 1 0],[0 0 1 1],c1);patch([0 1 1 1-e 1-e 0+e],[0 0 1 1-e 0+e 0+e],c2);patch([0 0 1 1-e 0+e 0+e],[0 1 1 1-e 1-e 0+e],c3);plot([0 1 1 0 0],[0 0 1 1 0]);disp('b');
  netsum : Sum  Inputs=2  SaturateOnIntegerOverflow=on  InputSameDT=on  OutDataTypeStr=Inherit: Same as first input
      mask Display: e = 0.06;c1 = [120 150 230]/255;c2 = ([120 150 230]+0)/383;c3 = ([120 150 230]+128)/383;patch([0 1 1 0],[0 0 1 1],c1);patch([0 1 1 1-e 1-e 0+e],[0 0 1 1-e 0+e 0+e],c2);patch([0 0 1 1-e 0+e 0+e],[0 1 1 1-e 1-e 0+e],c3);plot([0 1 1 0 0],[0 0 1 1 0]);disp('+');
  purelin : neural/Transfer Functions/purelin
      settings: SourceProductName=Deep Learning Toolbox
  "a{7}" : Outport  InitialOutput=[0;0;0;0;0]
      mask Display: e = 0.06;c1 = [120 230 180]/255;c2 = ([120 230 180]+0)/(256+128);c3 = ([120 230 180]+128)/(256+128);patch([0 1 1 0],[0 0 1 1],c1);patch([0 1 1 1-e 1-e 0+e],[0 0 1 1-e 0+e 0+e],c2);patch([0 0 1 1-e 0+e 0+e],[0 1 1 1-e 1-e 0+e],c3);plot([0 1 1 0 0],[0 0 1 1 0]);
  wires:
  purelin/1 -> "a{7}"/1
  netsum/1 -> purelin/1
  "b{7}"/1 -> netsum/2
  "LW{7,6}"/1("lz{7,6}") -> netsum/1
  Delays 1/1("pd{7,6}") -> "LW{7,6}"/1("ad{7,6}")
  "a{6} "/1 -> Delays 1/1("p{7}")

== system AcasXuClosedLoop_all_comp2017b/networks/Networks_m_6/AcasXu_3_6/Process Input 1 ==
  x : Inport  PortDimensions=5
      mask Display: e = 0.06;c1 = [120 230 180]/255;c2 = ([120 230 180]+0)/(256+128);c3 = ([120 230 180]+128)/(256+128);patch([0 1 1 0],[0 0 1 1],c1);patch([0 1 1 1-e 1-e 0+e],[0 0 1 1-e 0+e 0+e],c2);patch([0 0 1 1-e 0+e 0+e],[0 1 1 1-e 1-e 0+e],c3);plot([0 1 1 0 0],[0 0 1 1 0]);
  p : Outport  PortDimensions=5
      mask Display: e = 0.06;c1 = [120 230 180]/255;c2 = ([120 230 180]+0)/(256+128);c3 = ([120 230 180]+128)/(256+128);patch([0 1 1 0],[0 0 1 1],c1);patch([0 1 1 1-e 1-e 0+e],[0 0 1 1-e 0+e 0+e],c2);patch([0 0 1 1-e 0+e 0+e],[0 1 1 1-e 1-e 0+e],c3);plot([0 1 1 0 0],[0 0 1 1 0]);
  wires:
  x/1 -> p/1

== system AcasXuClosedLoop_all_comp2017b/networks/Networks_m_6/AcasXu_3_6/Process Output 1 ==
  a : Inport  PortDimensions=5
      mask Display: e = 0.06;c1 = [120 230 180]/255;c2 = ([120 230 180]+0)/(256+128);c3 = ([120 230 180]+128)/(256+128);patch([0 1 1 0],[0 0 1 1],c1);patch([0 1 1 1-e 1-e 0+e],[0 0 1 1-e 0+e 0+e],c2);patch([0 0 1 1-e 0+e 0+e],[0 1 1 1-e 1-e 0+e],c3);plot([0 1 1 0 0],[0 0 1 1 0]);
  y : Outport  PortDimensions=5
      mask Display: e = 0.06;c1 = [120 230 180]/255;c2 = ([120 230 180]+0)/(256+128);c3 = ([120 230 180]+128)/(256+128);patch([0 1 1 0],[0 0 1 1],c1);patch([0 1 1 1-e 1-e 0+e],[0 0 1 1-e 0+e 0+e],c2);patch([0 0 1 1-e 0+e 0+e],[0 1 1 1-e 1-e 0+e],c3);plot([0 1 1 0 0],[0 0 1 1 0]);
  wires:
  a/1 -> y/1

== system AcasXuClosedLoop_all_comp2017b/networks/Networks_m_6/AcasXu_4_6/Layer 1 ==
  "p{1}" : Inport  PortDimensions=5
      mask Display: e = 0.06;c1 = [120 230 180]/255;c2 = ([120 230 180]+0)/(256+128);c3 = ([120 230 180]+128)/(256+128);patch([0 1 1 0],[0 0 1 1],c1);patch([0 1 1 1-e 1-e 0+e],[0 0 1 1-e 0+e 0+e],c2);patch([0 0 1 1-e 0+e 0+e],[0 1 1 1-e 1-e 0+e],c3);plot([0 1 1 0 0],[0 0 1 1 0]);
  Delays 1 : Subsystem
      mask Display: e = 0.06;c1 = [120 150 230]/255;c2 = ([120 150 230]+0)/383;c3 = ([120 150 230]+128)/383;patch([0 1 1 0],[0 0 1 1],c1);patch([0 1 1 1-e 1-e 0+e],[0 0 1 1-e 0+e 0+e],c2);patch([0 0 1 1-e 0+e 0+e],[0 1 1 1-e 1-e 0+e],c3);plot([0 1 1 0 0],[0 0 1 1 0]);disp('0');
  "IW{1,1}" : Subsystem
      mask Display: e = 0.06;c1 = [120 150 230]/255;c2 = ([120 150 230]+0)/383;c3 = ([120 150 230]+128)/383;patch([0 1 1 0],[0 0 1 1],c1);patch([0 1 1 1-e 1-e 0+e],[0 0 1 1-e 0+e 0+e],c2);patch([0 0 1 1-e 0+e 0+e],[0 1 1 1-e 1-e 0+e],c3);plot([0 1 1 0 0],[0 0 1 1 0]);disp('W');
  "b{1}" : Constant  Value=[-0.3043270000000000141682221510563977062702178955078125;-0.0238544000000000012973178087349879206158220767974853515625;0.168115999999999987668530820883461274206638336181640625;-0.4819809999999999927666749499621801078319549560546875;-0.4534030000000000004689582056016661226749420166015625;0.2253090000000000092672536311511066742241382598876953125;-0.050409000000000002417177569213890819810330867767333984375;-0.07337069999999999703188535704612149856984615325927734375;-0.42685600000000001319477860306506045162677764892578125;0.085462399999999993926991237458423711359500885009765625;-0.152156000000000013461232128975098021328449249267578125;-0.190359000000000000429878355134860612452030181884765625;0.1590729999999999921822535497994977049529552459716796875;-0.2463859999999999939035433271783404052257537841796875;-0.016696099999999998442223869687950355000793933868408203125;-0.08507810000000000372200048559534479863941669464111328125;0.03069639999999999868673938863139483146369457244873046875;0.0715411999999999992372323731615324504673480987548828125;-0.265108999999999983554488380832481198012828826904296875;0.10195700000000000595701266092873993329703807830810546875;-0.0656071000000000015273116105163353495299816131591796875;0.025530799999999999327915389812915236689150333404541015625;-0.020137200000000000932320887159221456386148929595947265625;-0.56721600000000005348255172066274099051952362060546875;0.038778899999999998093880293481561238877475261688232421875;-0.0236961000000000011900258556352127925492823123931884765625;0.038043500000000000926814180957080679945647716522216796875;0.1429930000000000089865892505258671008050441741943359375;0.1990429999999999977067233203342766501009464263916015625;0.1421759999999999968256503279917524196207523345947265625;0.05759609999999999729869415432403911836445331573486328125;0.043190399999999996849542327481685788370668888092041015625;-0.321936999999999973187669866092619486153125762939453125;-0.139160000000000005915268275202834047377109527587890625;-0.50821799999999994756905152826220728456974029541015625;0.247572999999999987519316846373840235173702239990234375;-0.1334140000000000048085979642564780078828334808349609375;-0.51029899999999994708588246794533915817737579345703125;-0.01488860000000000009812151091637133504264056682586669921875;0.36600500000000002476241434123949147760868072509765625;-0.38602999999999998426147840291378088295459747314453125;-0.352376999999999995782928863263805396854877471923828125;-0.2181400000000000005684341886080801486968994140625;-0.0865853000000000039282355146497138775885105133056640625;0.35038999999999997925215211580507457256317138671875;-0.4179019999999999956941110212937928736209869384765625;-0.62205100000000002058442305497010238468647003173828125;-0.02125849999999999961897145794864627532660961151123046875;-0.5925380000000000091375795818748883903026580810546875;-0.0202103999999999998926636379792398656718432903289794921875]
      mask Display: e = 0.06;c1 = [120 150 230]/255;c2 = ([120 150 230]+0)/383;c3 = ([120 150 230]+128)/383;patch([0 1 1 0],[0 0 1 1],c1);patch([0 1 1 1-e 1-e 0+e],[0 0 1 1-e 0+e 0+e],c2);patch([0 0 1 1-e 0+e 0+e],[0 1 1 1-e 1-e 0+e],c3);plot([0 1 1 0 0],[0 0 1 1 0]);disp('b');
  netsum : Sum  Inputs=2  SaturateOnIntegerOverflow=on  InputSameDT=on  OutDataTypeStr=Inherit: Same as first input
      mask Display: e = 0.06;c1 = [120 150 230]/255;c2 = ([120 150 230]+0)/383;c3 = ([120 150 230]+128)/383;patch([0 1 1 0],[0 0 1 1],c1);patch([0 1 1 1-e 1-e 0+e],[0 0 1 1-e 0+e 0+e],c2);patch([0 0 1 1-e 0+e 0+e],[0 1 1 1-e 1-e 0+e],c3);plot([0 1 1 0 0],[0 0 1 1 0]);disp('+');
  poslin : neural/Transfer Functions/poslin
      settings: SourceProductName=Deep Learning Toolbox  SourceProductBaseCode=NN
  "a{1}" : Outport  InitialOutput=[0;0;0;0;0;0;0;0;0;0;0;0;0;0;0;0;0;0;0;0;0;0;0;0;0;0;0;0;0;0;0;0;0;0;0;0;0;0;0;0;0;0;0;0;0;0;0;0;0;0]
      mask Display: e = 0.06;c1 = [120 230 180]/255;c2 = ([120 230 180]+0)/(256+128);c3 = ([120 230 180]+128)/(256+128);patch([0 1 1 0],[0 0 1 1],c1);patch([0 1 1 1-e 1-e 0+e],[0 0 1 1-e 0+e 0+e],c2);patch([0 0 1 1-e 0+e 0+e],[0 1 1 1-e 1-e 0+e],c3);plot([0 1 1 0 0],[0 0 1 1 0]);
  wires:
  poslin/1 -> "a{1}"/1
  netsum/1 -> poslin/1
  "b{1}"/1 -> netsum/2
  "IW{1,1}"/1("iz{1,1}") -> netsum/1
  Delays 1/1("pd{1,1}") -> "IW{1,1}"/1("pd{1,1}")
  "p{1}"/1 -> Delays 1/1("p{1}")

== system AcasXuClosedLoop_all_comp2017b/networks/Networks_m_6/AcasXu_4_6/Layer 2 ==
  "a{1} " : Inport  PortDimensions=50
      mask Display: e = 0.06;c1 = [120 230 180]/255;c2 = ([120 230 180]+0)/(256+128);c3 = ([120 230 180]+128)/(256+128);patch([0 1 1 0],[0 0 1 1],c1);patch([0 1 1 1-e 1-e 0+e],[0 0 1 1-e 0+e 0+e],c2);patch([0 0 1 1-e 0+e 0+e],[0 1 1 1-e 1-e 0+e],c3);plot([0 1 1 0 0],[0 0 1 1 0]);
  Delays 1 : Subsystem
      mask Display: e = 0.06;c1 = [120 150 230]/255;c2 = ([120 150 230]+0)/383;c3 = ([120 150 230]+128)/383;patch([0 1 1 0],[0 0 1 1],c1);patch([0 1 1 1-e 1-e 0+e],[0 0 1 1-e 0+e 0+e],c2);patch([0 0 1 1-e 0+e 0+e],[0 1 1 1-e 1-e 0+e],c3);plot([0 1 1 0 0],[0 0 1 1 0]);disp('0');
  "LW{2,1}" : Subsystem
      mask Display: e = 0.06;c1 = [120 150 230]/255;c2 = ([120 150 230]+0)/383;c3 = ([120 150 230]+128)/383;patch([0 1 1 0],[0 0 1 1],c1);patch([0 1 1 1-e 1-e 0+e],[0 0 1 1-e 0+e 0+e],c2);patch([0 0 1 1-e 0+e 0+e],[0 1 1 1-e 1-e 0+e],c3);plot([0 1 1 0 0],[0 0 1 1 0]);disp('W');
  "b{2}" : Constant  Value=[-0.0299640000000000010504930259003231185488402843475341796875;0.4516430000000000166693325809319503605365753173828125;-0.218150000000000010569323194431490264832973480224609375;0.11300399999999999334221456592786125838756561279296875;0.76343700000000003225153477615094743669033050537109375;-0.53257799999999999585043042316101491451263427734375;0.10861200000000000021049828546892968006432056427001953125;-0.01558400000000000062971849956738878972828388214111328125;-0.0268861999999999989885424156454973854124546051025390625;-0.2047650000000000025668356329333619214594364166259765625;0.5264100000000000445510295321582816541194915771484375;0.63073999999999996735056129182339645922183990478515625;-0.5641599999999999948130380289512686431407928466796875;0.1327329999999999898818714427761733531951904296875;-0.1656430000000000124504850873563555069267749786376953125;-0.177769000000000010341949518988258205354213714599609375;-0.0225980999999999994376498335668657091446220874786376953125;-0.357563000000000019706902776306378655135631561279296875;1.074079999999999923687710179365240037441253662109375;-0.2076699999999999934896521835980820469558238983154296875;-1.27259000000000010999201549566350877285003662109375;0.265390999999999988023802188763511367142200469970703125;0.96386300000000002530242682041716761887073516845703125;1.0210999999999998966160319469054229557514190673828125;-0.60398899999999999810285089552053250372409820556640625;0.2071800000000000030464519795714295469224452972412109375;0.30389100000000002221867134721833281219005584716796875;-0.0207310999999999988341325973806306137703359127044677734375;0.15072900000000000186872739504906348884105682373046875;-0.70596300000000000718358705853461287915706634521484375;-0.25036199999999997345412339200265705585479736328125;0.59141600000000005277200898490264080464839935302734375;-0.59254300000000004189359970041550695896148681640625;1.0614699999999999135980033315718173980712890625;-0.406308999999999975738518287471379153430461883544921875;0.0238720000000000008577583088253959431312978267669677734375;-0.012841999999999999249045146143544116057455539703369140625;0.68951399999999996026645021629519760608673095703125;-0.314454000000000011283418643870390951633453369140625;-0.67384900000000003128519665551721118390560150146484375;-0.0167743999999999983396836711335708969272673130035400390625;-0.1203100000000000002753353101070388220250606536865234375;-0.052208499999999997964739151257163030095398426055908203125;-0.66984100000000001973177177205798216164112091064453125;0.1798260000000000136122224603241193108260631561279296875;0.55144999999999999573674358543939888477325439453125;-0.01235960000000000010011991236069661681540310382843017578125;0.82090899999999999980815346134477294981479644775390625;-0.020357699999999999407318540534106432460248470306396484375;0.10281300000000000161026747491632704623043537139892578125]
      mask Display: e = 0.06;c1 = [120 150 230]/255;c2 = ([120 150 230]+0)/383;c3 = ([120 150 230]+128)/383;patch([0 1 1 0],[0 0 1 1],c1);patch([0 1 1 1-e 1-e 0+e],[0 0 1 1-e 0+e 0+e],c2);patch([0 0 1 1-e 0+e 0+e],[0 1 1 1-e 1-e 0+e],c3);plot([0 1 1 0 0],[0 0 1 1 0]);disp('b');
  netsum : Sum  Inputs=2  SaturateOnIntegerOverflow=on  InputSameDT=on  OutDataTypeStr=Inherit: Same as first input
      mask Display: e = 0.06;c1 = [120 150 230]/255;c2 = ([120 150 230]+0)/383;c3 = ([120 150 230]+128)/383;patch([0 1 1 0],[0 0 1 1],c1);patch([0 1 1 1-e 1-e 0+e],[0 0 1 1-e 0+e 0+e],c2);patch([0 0 1 1-e 0+e 0+e],[0 1 1 1-e 1-e 0+e],c3);plot([0 1 1 0 0],[0 0 1 1 0]);disp('+');
  poslin : neural/Transfer Functions/poslin
      settings: SourceProductName=Deep Learning Toolbox  SourceProductBaseCode=NN
  "a{2}" : Outport  InitialOutput=[0;0;0;0;0;0;0;0;0;0;0;0;0;0;0;0;0;0;0;0;0;0;0;0;0;0;0;0;0;0;0;0;0;0;0;0;0;0;0;0;0;0;0;0;0;0;0;0;0;0]
      mask Display: e = 0.06;c1 = [120 230 180]/255;c2 = ([120 230 180]+0)/(256+128);c3 = ([120 230 180]+128)/(256+128);patch([0 1 1 0],[0 0 1 1],c1);patch([0 1 1 1-e 1-e 0+e],[0 0 1 1-e 0+e 0+e],c2);patch([0 0 1 1-e 0+e 0+e],[0 1 1 1-e 1-e 0+e],c3);plot([0 1 1 0 0],[0 0 1 1 0]);
  wires:
  poslin/1 -> "a{2}"/1
  netsum/1 -> poslin/1
  "b{2}"/1 -> netsum/2
  "LW{2,1}"/1("lz{2,1}") -> netsum/1
  Delays 1/1("pd{2,1}") -> "LW{2,1}"/1("ad{2,1}")
  "a{1} "/1 -> Delays 1/1("p{2}")
